simulink model "MCVT_Integration"  (R2020a)
settings: Solver=ode8  StartTime=0.0  StopTime=T_end  FixedStep=dt  RelTol=1e-3  EnableMultiTasking=on
values from neighbouring files (symbols this model uses):
  assumed_power_voltage = 1.25e2    (= 125)    [MCVT_Input_File.m]    (also in MCVT_Run_QuickCheck.m)
  battery_power_toggle = 1    [MCVT_Input_File.m]    (also in MCVT_Run_QuickCheck.m)
  battery_trickle_charge_param = 0.1    [MCVT_Input_File.m]    (also in MCVT_Run_QuickCheck.m)
  battery_trickle_leakage_param = 0.01    [MCVT_Input_File.m]    (also in MCVT_Run_QuickCheck.m)
  damage_case_id = 3    [MCVT_Input_File.m]    (also in MCVT_Run_QuickCheck.m = 1)
  dt = 1/fs    (= 0.001)    [MCVT_Run_QuickCheck.m]    (also in MCVT_Sim_Set.m)
  dt_2 = 1/fs_2    (= 0.001)    [MCVT_Run_QuickCheck.m]    (also in MCVT_Sim_Set.m)
  dt_3 = 1/fs_3    (= 0.001)    [MCVT_Run_QuickCheck.m]    (also in MCVT_Sim_Set.m)
  dt_5 = 1/fs_5    (= 0.005)    [MCVT_Run_QuickCheck.m]    (also in MCVT_Sim_Set.m)
  dt_8 = 1/fs_8    (= 0.05)    [MCVT_Run_QuickCheck.m]    (also in MCVT_Sim_Set.m)
  dt_9 = 1/fs_9    (= 0.001)    [MCVT_Run_QuickCheck.m]    (also in MCVT_Sim_Set.m)
  dust_met_duration = 3    [MCVT_Input_File.m]    (also in MCVT_Run_QuickCheck.m = 2)
  ECLSS_indoor_set_temperature = 298.15    [MCVT_Input_File.m]    (also in MCVT_Run_QuickCheck.m)
  energy_store_max_o = 150    [MCVT_Input_File.m]    (also in MCVT_Run_QuickCheck.m)
  energy_store_o = 120    [MCVT_Input_File.m]    (also in MCVT_Run_QuickCheck.m)
  fs = 1000    [MCVT_Run_QuickCheck.m]    (also in MCVT_Sim_Set.m)
  gain_charge_param = 1    [MCVT_Input_File.m]    (also in MCVT_Run_QuickCheck.m)
  ground_temperature = 373    [MCVT_Habitat_Design_Input_File.m]    (also in MCVT_Run_QuickCheck.m)
  I_max_charge = 800    [MCVT_Input_File.m]    (also in MCVT_Run_QuickCheck.m)
  I_max_discharge = 800    [MCVT_Input_File.m]    (also in MCVT_Run_QuickCheck.m)
  initial_pressure = 1e5    (= 100000)    [MCVT_Input_File.m]    (also in MCVT_Run_QuickCheck.m)
  initial_temperature = 295    [MCVT_Input_File.m]    (also in MCVT_Run_QuickCheck.m)
  interior_leak_rate = 0.00415    [MCVT_Input_File.m]    (also in MCVT_Run_QuickCheck.m)
  launch_dust_accum_start_time = 10    [MCVT_Input_File.m]    (also in MCVT_Run_QuickCheck.m = 2)
  launch_dust_accum_stop_time = 12    [MCVT_Input_File.m]    (also in MCVT_Run_QuickCheck.m = 3)
  meteorite_impact_time = 2    [MCVT_Input_File.m]    (also in MCVT_Run_QuickCheck.m = 1)
  nuclear_power_toggle = 1    [MCVT_Input_File.m]    (also in MCVT_Run_QuickCheck.m)
  print_interval = 30    [MCVT_Input_File.m]
  SOC_lower_thres = 0.5    [MCVT_Input_File.m]    (also in MCVT_Run_QuickCheck.m)
  SOC_upper_thres = 0.9    [MCVT_Input_File.m]    (also in MCVT_Run_QuickCheck.m)
  solar_power_toggle = 1    [MCVT_Input_File.m]    (also in MCVT_Run_QuickCheck.m)
  space_temperature = 4    [MCVT_Habitat_Design_Input_File.m]    (also in MCVT_Run_QuickCheck.m)
  Start_angle = 90    [MCVT_Input_File.m]    (also in MCVT_Run_QuickCheck.m)
undefined symbols (not defined in the file or in its folder): A_flow_restrictor, ACTIVITY_LOOKUP_TABLE, air, Aref, Bmap, Bmap2, Bmap3, comms, DiscIntNeverNeededParam, DmgSPL, dust_parameters, ECLSS_dust_damage_repair_time, ECLSS_heater_capacity, ECLSS_IPS_restrictor, ECLSS_IPS_setpointPressure, ECLSS_paint_damage_repair_time, ECLSS_radiatorLoop_discreteNumber, ECLSS_Vst, emptyActivity, expected_heat_add, FI, habitat, HIEM2SMM, HIEM2STM, HIEMDamageElements, impact_numbering, initial_SPLT_damage_index, input_air, input_Cond, input_CP, input_discretization, input_EV, input_Evap, input_radiatorLoop, K_ECLSS_dust_single, K_ECLSS_paint_single, K_Structure_damage_single, Kref, kW_to_W, meteoroidDustDep, mFRF, ndelays, nominalDustDep, noverlap, nuclear_toggle_gain, output_limits, panel_paint0, Ps0, reference_temperature, RepairTime_ECLSS_factor, Sensor_Location_SMM, SMM_T0, SMM_T1, SMM_T2, SMM_T3, SMM_T4, SMM_T4_0, SMM_T4_1, SMM_T4_2, solar_FDD_K ... (+73)
libraries: neural x350, simulink x39, xpcutilitieslib x7, xpcrtudplib x7, dspstat3 x1
(part 18 of 43)

== system MCVT_Integration/Sys2 - Structural System/Sys2_Structural_System/Fault Detection and Diagnosis (FDD) with Binary Classifer/Pattern Recognition Neural Network/Segment 5/Process Output 1 ==
  a : Inport  PortDimensions=2
      mask Display: e = 0.06;c1 = [120 230 180]/255;c2 = ([120 230 180]+0)/(256+128);c3 = ([120 230 180]+128)/(256+128);patch([0 1 1 0],[0 0 1 1],c1);patch([0 1 1 1-e 1-e 0+e],[0 0 1 1-e 0+e 0+e],c2);patch([0 0 1 1-e 0+e 0+e],[0 1 1 1-e 1-e 0+e],c3);plot([0 1 1 0 0],[0 0 1 1 0]);
  y : Outport  PortDimensions=2
      mask Display: e = 0.06;c1 = [120 230 180]/255;c2 = ([120 230 180]+0)/(256+128);c3 = ([120 230 180]+128)/(256+128);patch([0 1 1 0],[0 0 1 1],c1);patch([0 1 1 1-e 1-e 0+e],[0 0 1 1-e 0+e 0+e],c2);patch([0 0 1 1-e 0+e 0+e],[0 1 1 1-e 1-e 0+e],c3);plot([0 1 1 0 0],[0 0 1 1 0]);
  wires:
  a/1 -> y/1

== system MCVT_Integration/Sys2 - Structural System/Sys2_Structural_System/Fault Detection and Diagnosis (FDD) with Binary Classifer/Subsystem/Enabled Subsystem3/Pattern Recognition Neural Network1 ==
  Input : Inport  PortDimensions=45  SampleTime=0.001
      mask Display: e = 0.06;c1 = [120 230 180]/255;c2 = ([120 230 180]+0)/(256+128);c3 = ([120 230 180]+128)/(256+128);patch([0 1 1 0],[0 0 1 1],c1);patch([0 1 1 1-e 1-e 0+e],[0 0 1 1-e 0+e 0+e],c2);patch([0 0 1 1-e 0+e 0+e],[0 1 1 1-e 1-e 0+e],c3);plot([0 1 1 0 0],[0 0 1 1 0]);
  Layer 1 : Subsystem
      mask Display: e = 0.06;c1 = [120 150 230]/255;c2 = ([120 150 230]+0)/383;c3 = ([120 150 230]+128)/383;patch([0 1 1 0],[0 0 1 1],c1);patch([0 1 1 1-e 1-e 0+e],[0 0 1 1-e 0+e 0+e],c2);patch([0 0 1 1-e 0+e 0+e],[0 1 1 1-e 1-e 0+e],c3);plot([0 1 1 0 0],[0 0 1 1 0]);
  Layer 2 : Subsystem
      mask Display: e = 0.06;c1 = [120 150 230]/255;c2 = ([120 150 230]+0)/383;c3 = ([120 150 230]+128)/383;patch([0 1 1 0],[0 0 1 1],c1);patch([0 1 1 1-e 1-e 0+e],[0 0 1 1-e 0+e 0+e],c2);patch([0 0 1 1-e 0+e 0+e],[0 1 1 1-e 1-e 0+e],c3);plot([0 1 1 0 0],[0 0 1 1 0]);
  Layer 3 : Subsystem
      mask Display: e = 0.06;c1 = [120 150 230]/255;c2 = ([120 150 230]+0)/383;c3 = ([120 150 230]+128)/383;patch([0 1 1 0],[0 0 1 1],c1);patch([0 1 1 1-e 1-e 0+e],[0 0 1 1-e 0+e 0+e],c2);patch([0 0 1 1-e 0+e 0+e],[0 1 1 1-e 1-e 0+e],c3);plot([0 1 1 0 0],[0 0 1 1 0]);
  Layer 4 : Subsystem
      mask Display: e = 0.06;c1 = [120 150 230]/255;c2 = ([120 150 230]+0)/383;c3 = ([120 150 230]+128)/383;patch([0 1 1 0],[0 0 1 1],c1);patch([0 1 1 1-e 1-e 0+e],[0 0 1 1-e 0+e 0+e],c2);patch([0 0 1 1-e 0+e 0+e],[0 1 1 1-e 1-e 0+e],c3);plot([0 1 1 0 0],[0 0 1 1 0]);
  Process Input 1 : Subsystem
      mask Display: e = 0.06;c1 = [120 150 230]/255;c2 = ([120 150 230]+0)/383;c3 = ([120 150 230]+128)/383;patch([0 1 1 0],[0 0 1 1],c1);patch([0 1 1 1-e 1-e 0+e],[0 0 1 1-e 0+e 0+e],c2);patch([0 0 1 1-e 0+e 0+e],[0 1 1 1-e 1-e 0+e],c3);plot([0 1 1 0 0],[0 0 1 1 0]);
  Process Output 1 : Subsystem
      mask Display: e = 0.06;c1 = [120 150 230]/255;c2 = ([120 150 230]+0)/383;c3 = ([120 150 230]+128)/383;patch([0 1 1 0],[0 0 1 1],c1);patch([0 1 1 1-e 1-e 0+e],[0 0 1 1-e 0+e 0+e],c2);patch([0 0 1 1-e 0+e 0+e],[0 1 1 1-e 1-e 0+e],c3);plot([0 1 1 0 0],[0 0 1 1 0]);
  Output : Outport  InitialOutput=[0;0;0;0;0]
      mask Display: e = 0.06;c1 = [120 230 180]/255;c2 = ([120 230 180]+0)/(256+128);c3 = ([120 230 180]+128)/(256+128);patch([0 1 1 0],[0 0 1 1],c1);patch([0 1 1 1-e 1-e 0+e],[0 0 1 1-e 0+e 0+e],c2);patch([0 0 1 1-e 0+e 0+e],[0 1 1 1-e 1-e 0+e],c3);plot([0 1 1 0 0],[0 0 1 1 0]);
  wires:
  Input/1 -> Process Input 1/1(x)
  Process Output 1/1(y) -> Output/1
  Process Input 1/1(p) -> Layer 1/1("p{1}")
  Layer 1/1("a{1}") -> [feedback1]
  [feedback1] -> Layer 2/1("a{1} ")
  Layer 2/1("a{2}") -> [feedback2]
  [feedback2] -> Layer 3/1("a{2} ")
  Layer 3/1("a{3}") -> [feedback3]
  [feedback3] -> Layer 4/1("a{3} ")
  Layer 4/1("a{4}") -> Process Output 1/1(a)

== system MCVT_Integration/Sys2 - Structural System/Sys2_Structural_System/Structural Thermal Model 2.2/STM Temperature Sensors/ Resistance Temperature Detector/RTD with Self-heat Error ==
  Resistance Change with Error : Inport
  " Current in Circuit" : Constant  Value=0.001
  MATLAB Function : MATLAB Function  TreatAsAtomicUnit=on
  Voltage Output with Error : Outport  VectorParamsAs1DForOutWhenUnconnected=off
  wires:
  Resistance Change with Error/1 -> MATLAB Function/2(R)
  MATLAB Function/1(U) -> Voltage Output with Error/1
  " Current in Circuit"/1 -> MATLAB Function/1(I)

== system MCVT_Integration/Sys2 - Structural System/Sys2_Structural_System/Structural Thermal Model 2.2/STM Temperature Sensors/ Resistance Temperature Detector/RTD without Self-heat Error ==
  Resistance Change without Error : Inport
  " Current in Circuit" : Constant  Value=0.001
  MATLAB Function : MATLAB Function  TreatAsAtomicUnit=on
  Current in Circuit : Outport  VectorParamsAs1DForOutWhenUnconnected=off
  Voltage Output without Error : Outport  Port=2  VectorParamsAs1DForOutWhenUnconnected=off
  wires:
  Resistance Change without Error/1 -> MATLAB Function/2(R)
  MATLAB Function/2(U) -> Voltage Output without Error/1
  MATLAB Function/1(I) -> Current in Circuit/1
  " Current in Circuit"/1 -> MATLAB Function/1("I ")

== system MCVT_Integration/Sys2 - Structural System/Sys2_Structural_System/Structural Thermal Model 2.2/STM Temperature Sensors/ Resistance Temperature Detector/Self-heat Calculation ==
  note: Power = I 2 *R
  Real Temperature : Inport
  Resistance Change without Error : Inport  Port=2
  Current in Circuit : Inport  Port=3
  "  T vs R " : MATLAB Function  TreatAsAtomicUnit=on
  Add1 : Sum
  Divide1 : Product  Inputs=*/
  Gain : Gain  Gain=1000
  Product : Product
  Self Heating Constant (mW/℃) : Constant  Value=50
  Square : Math  Operator=square  SignedPower=on  SaturateOnIntegerOverflow=on
  Resistance Change with Error : Outport  VectorParamsAs1DForOutWhenUnconnected=off
  Self-heat Error : Outport  Port=2  VectorParamsAs1DForOutWhenUnconnected=off
  wires:
  Self Heating Constant (mW/℃)/1 -> Divide1/2
  Product/1 -> Divide1/1
  Add1/1 -> "  T vs R "/1(T)
  Divide1/1 -> Self-heat Error/1, Add1/2
  Resistance Change without Error/1 -> Product/1
  "  T vs R "/1(R) -> Resistance Change with Error/1
  Real Temperature/1 -> Add1/1
  Gain/1 -> Square/1
  Square/1 -> Product/2
  Current in Circuit/1 -> Gain/1

== system MCVT_Integration/Sys2 - Structural System/Sys2_Structural_System/Structural Thermal Model 2.2/STM Temperature Sensors/ Resistance Temperature Detector/Switch ON//OFF Control Block ==
  In1 : Inport
  In2 : Inport  Port=2
  Multiport Switch1 : MultiPortSwitch  Inputs=2
  Switch OFF : Subsystem
  Switch ON : Subsystem
  Out1 : Outport  VectorParamsAs1DForOutWhenUnconnected=off
  wires:
  Multiport Switch1/1 -> Out1/1
  In2/1 -> Switch ON/1(In1), Switch OFF/1(In1)
  In1/1 -> Multiport Switch1/1
  Switch ON/1(ON) -> Multiport Switch1/2
  Switch OFF/1(OFF) -> Multiport Switch1/3

== system MCVT_Integration/Sys2 - Structural System/Sys2_Structural_System/Structural Thermal Model 2.2/STM Temperature Sensors/ Resistance Temperature Detector1/RTD with Self-heat Error ==
  Resistance Change with Error : Inport
  " Current in Circuit" : Constant  Value=0.001
  MATLAB Function : MATLAB Function  TreatAsAtomicUnit=on
  Voltage Output with Error : Outport  VectorParamsAs1DForOutWhenUnconnected=off
  wires:
  " Current in Circuit"/1 -> MATLAB Function/1(I)
  MATLAB Function/1(U) -> Voltage Output with Error/1
  Resistance Change with Error/1 -> MATLAB Function/2(R)

== system MCVT_Integration/Sys2 - Structural System/Sys2_Structural_System/Structural Thermal Model 2.2/STM Temperature Sensors/ Resistance Temperature Detector1/RTD without Self-heat Error ==
  Resistance Change without Error : Inport
  " Current in Circuit" : Constant  Value=0.001
  MATLAB Function : MATLAB Function  TreatAsAtomicUnit=on
  Current in Circuit : Outport  VectorParamsAs1DForOutWhenUnconnected=off
  Voltage Output without Error : Outport  Port=2  VectorParamsAs1DForOutWhenUnconnected=off
  wires:
  " Current in Circuit"/1 -> MATLAB Function/1("I ")
  MATLAB Function/1(I) -> Current in Circuit/1
  MATLAB Function/2(U) -> Voltage Output without Error/1
  Resistance Change without Error/1 -> MATLAB Function/2(R)

== system MCVT_Integration/Sys2 - Structural System/Sys2_Structural_System/Structural Thermal Model 2.2/STM Temperature Sensors/ Resistance Temperature Detector1/Self-heat Calculation ==
  note: Power = I 2 *R
  Real Temperature : Inport
  Resistance Change without Error : Inport  Port=2
  Current in Circuit : Inport  Port=3
  "  T vs R " : MATLAB Function  TreatAsAtomicUnit=on
  Add1 : Sum
  Divide1 : Product  Inputs=*/
  Gain : Gain  Gain=1000
  Product : Product
  Self Heating Constant (mW/℃) : Constant  Value=50
  Square : Math  Operator=square  SignedPower=on  SaturateOnIntegerOverflow=on
  Resistance Change with Error : Outport  VectorParamsAs1DForOutWhenUnconnected=off
  Self-heat Error : Outport  Port=2  VectorParamsAs1DForOutWhenUnconnected=off
  wires:
  Current in Circuit/1 -> Gain/1
  Square/1 -> Product/2
  Gain/1 -> Square/1
  Real Temperature/1 -> Add1/1
  "  T vs R "/1(R) -> Resistance Change with Error/1
  Resistance Change without Error/1 -> Product/1
  Divide1/1 -> Add1/2, Self-heat Error/1
  Add1/1 -> "  T vs R "/1(T)
  Product/1 -> Divide1/1
  Self Heating Constant (mW/℃)/1 -> Divide1/2

== system MCVT_Integration/Sys2 - Structural System/Sys2_Structural_System/Structural Thermal Model 2.2/STM Temperature Sensors/ Resistance Temperature Detector1/Switch ON//OFF Control Block ==
  In1 : Inport
  In2 : Inport  Port=2
  Multiport Switch1 : MultiPortSwitch  Inputs=2
  Switch OFF : Subsystem
  Switch ON : Subsystem
  Out1 : Outport  VectorParamsAs1DForOutWhenUnconnected=off
  wires:
  Switch OFF/1(OFF) -> Multiport Switch1/3
  Switch ON/1(ON) -> Multiport Switch1/2
  In1/1 -> Multiport Switch1/1
  In2/1 -> Switch OFF/1(In1), Switch ON/1(In1)
  Multiport Switch1/1 -> Out1/1

== system MCVT_Integration/Sys2 - Structural System/Sys2_Structural_System/Structural Thermal Model 2.2/STM Temperature Sensors/ Resistance Temperature Detector2/RTD with Self-heat Error ==
  Resistance Change with Error : Inport
  " Current in Circuit" : Constant  Value=0.001
  MATLAB Function : MATLAB Function  TreatAsAtomicUnit=on
  Voltage Output with Error : Outport  VectorParamsAs1DForOutWhenUnconnected=off
  wires:
  Resistance Change with Error/1 -> MATLAB Function/2(R)
  MATLAB Function/1(U) -> Voltage Output with Error/1
  " Current in Circuit"/1 -> MATLAB Function/1(I)

== system MCVT_Integration/Sys2 - Structural System/Sys2_Structural_System/Structural Thermal Model 2.2/STM Temperature Sensors/ Resistance Temperature Detector2/RTD without Self-heat Error ==
  Resistance Change without Error : Inport
  " Current in Circuit" : Constant  Value=0.001
  MATLAB Function : MATLAB Function  TreatAsAtomicUnit=on
  Current in Circuit : Outport  VectorParamsAs1DForOutWhenUnconnected=off
  Voltage Output without Error : Outport  Port=2  VectorParamsAs1DForOutWhenUnconnected=off
  wires:
  Resistance Change without Error/1 -> MATLAB Function/2(R)
  MATLAB Function/2(U) -> Voltage Output without Error/1
  MATLAB Function/1(I) -> Current in Circuit/1
  " Current in Circuit"/1 -> MATLAB Function/1("I ")

== system MCVT_Integration/Sys2 - Structural System/Sys2_Structural_System/Structural Thermal Model 2.2/STM Temperature Sensors/ Resistance Temperature Detector2/Self-heat Calculation ==
  note: Power = I 2 *R
  Real Temperature : Inport
  Resistance Change without Error : Inport  Port=2
  Current in Circuit : Inport  Port=3
  "  T vs R " : MATLAB Function  TreatAsAtomicUnit=on
  Add1 : Sum
  Divide1 : Product  Inputs=*/
  Gain : Gain  Gain=1000
  Product : Product
  Self Heating Constant (mW/℃) : Constant  Value=50
  Square : Math  Operator=square  SignedPower=on  SaturateOnIntegerOverflow=on
  Resistance Change with Error : Outport  VectorParamsAs1DForOutWhenUnconnected=off
  Self-heat Error : Outport  Port=2  VectorParamsAs1DForOutWhenUnconnected=off
  wires:
  Self Heating Constant (mW/℃)/1 -> Divide1/2
  Product/1 -> Divide1/1
  Add1/1 -> "  T vs R "/1(T)
  Divide1/1 -> Self-heat Error/1, Add1/2
  Resistance Change without Error/1 -> Product/1
  "  T vs R "/1(R) -> Resistance Change with Error/1
  Real Temperature/1 -> Add1/1
  Gain/1 -> Square/1
  Square/1 -> Product/2
  Current in Circuit/1 -> Gain/1

== system MCVT_Integration/Sys2 - Structural System/Sys2_Structural_System/Structural Thermal Model 2.2/STM Temperature Sensors/ Resistance Temperature Detector2/Switch ON//OFF Control Block ==
  In1 : Inport
  In2 : Inport  Port=2
  Multiport Switch1 : MultiPortSwitch  Inputs=2
  Switch OFF : Subsystem
  Switch ON : Subsystem
  Out1 : Outport  VectorParamsAs1DForOutWhenUnconnected=off
  wires:
  Multiport Switch1/1 -> Out1/1
  In2/1 -> Switch ON/1(In1), Switch OFF/1(In1)
  In1/1 -> Multiport Switch1/1
  Switch ON/1(ON) -> Multiport Switch1/2
  Switch OFF/1(OFF) -> Multiport Switch1/3

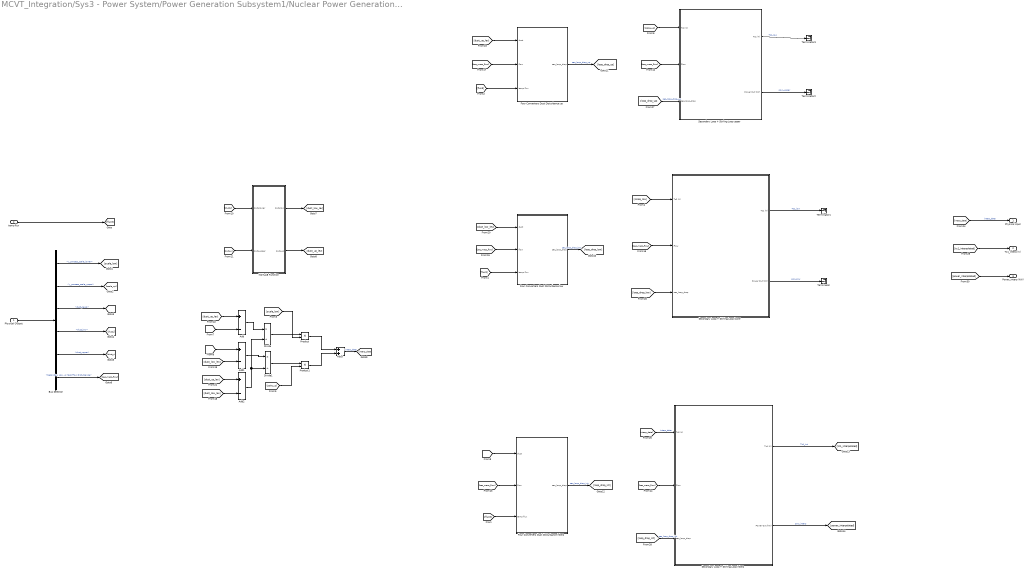
== system MCVT_Integration/Sys3 - Power System/Power Generation Subsystem1/Nuclear Power Generation/SP-100 Power Generation/	Power Generation/Interpolation ==
  Physical Output : Inport
  temp flux : Inport  Port=2
  Add : Sum  Inputs=+-
  Add1 : Sum  Inputs=+-
  Add2 : Sum  Inputs=+-
  Add3 : Sum
  Bus Selector : BusSelector  OutputSignals=y_process_scale_lower,y_process_scale_upper,dust_level,dust_low,dust_upper,Secondary Loop Mass Flow Disturbance
  Divide : Product  Inputs=*/
  Divide1 : Product  Inputs=*/
  Four Converters Dust Disturbance interp : Subsystem
  Four Converters Dust Disturbance low : Subsystem
  Four Converters Dust Disturbance up : Subsystem
  MATLAB Function : MATLAB Function  TreatAsAtomicUnit=on
  Product : Product
  Product1 : Product
  Secondary Loop + Stirling Loop interp : Subsystem
  Secondary Loop + Stirling Loop lower : Subsystem
  Secondary Loop + Stirling Loop upper : Subsystem
  Terminator{,1..3} (4 alike) : Terminator
  Physical Input : Outport
  Tc1_interp (K) : Outport  Port=2
  Power_interp (kW) : Outport  Port=3
  wires:
  Product/1 -> Add3/1
  [dust_l] -> MATLAB Function/1(dust_lower)
  Add1/1 -> Divide1/1
  [dust_u] -> MATLAB Function/2(dust_upper)
  Product1/1 -> Add3/2
  [interp_data] -> Physical Input/1  "interp_data"
  Divide1/1 -> Product1/1
  Divide/1 -> Product/1
  Add/1 -> Divide/1
  [dust_low_lev] -> Add2/2
  [scale_up] -> Product1/2
  [scale_low] -> Product/2
  Physical Output/1 -> Bus Selector/1
  Add2/1 -> Divide/2, Divide1/2
  [dust_up_lev] -> Add2/1
  [d_level] -> Add/2
  [sec_mass_flow] -> Secondary Loop + Stirling Loop upper/2(Flow)
  Secondary Loop + Stirling Loop interp/1(Tc1 (K)) -> [tc1_interpolated]  "Tc1_int"
  Secondary Loop + Stirling Loop interp/2(Power Out (kW)) -> [power_interpolated]  "pow_interp"
  Secondary Loop + Stirling Loop lower/2(Power Out (kW)) -> Terminator/1  "pow_low"
  Secondary Loop + Stirling Loop lower/1(Tc1 (K)) -> Terminator1/1  "Tc1_low"
  Secondary Loop + Stirling Loop upper/2(Power Out (kW)) -> Terminator2/1  "pow_upper"
  Secondary Loop + Stirling Loop upper/1(Tc1 (K)) -> Terminator3/1  "Tc1_low"
  [flux3] -> Four Converters Dust Disturbance interp/3(temp flux)
  Four Converters Dust Disturbance low/1(sec_loop_drop) -> [loop_drop_low]  "sec_loop_drop_low"
  [loop_drop_up] -> Secondary Loop + Stirling Loop upper/3(sec_loop_drop)  "sec_loop_drop_up"
  [loop_drop_int] -> Secondary Loop + Stirling Loop interp/3(sec_loop_drop)  "sec_loop_drop_int"
  temp flux/1 -> [flux3]
  [flux3] -> Four Converters Dust Disturbance low/3(temp flux)
  [flux3] -> Four Converters Dust Disturbance up/3(temp flux)
  Bus Selector/1 -> [scale_low]  "<y_process_scale_lower>"
  [scale_low] -> Secondary Loop + Stirling Loop lower/1(Tc2 (K))
  Bus Selector/2 -> [scale_up]  "<y_process_scale_upper>"
  [scale_up] -> Secondary Loop + Stirling Loop upper/1(Tc2 (K))
  Bus Selector/3 -> [d_level]  "<dust_level>"
  [d_level] -> Four Converters Dust Disturbance interp/1(dust)
  [d_level] -> Add1/1
  Bus Selector/4 -> [dust_l]  "<dust_low>"
  Bus Selector/5 -> [dust_u]  "<dust_upper>"
  Bus Selector/6 -> [sec_mass_flow]  "<Secondary Loop Mass Flow Disturbance>"
  [sec_mass_flow] -> Four Converters Dust Disturbance interp/2(flow)
  [sec_mass_flow] -> Secondary Loop + Stirling Loop interp/2(Flow)
  [sec_mass_flow] -> Four Converters Dust Disturbance low/2(flow)
  [sec_mass_flow] -> Secondary Loop + Stirling Loop lower/2(Flow)
  [sec_mass_flow] -> Four Converters Dust Disturbance up/2(flow)
  MATLAB Function/1(dust_low) -> [dust_low_lev]
  [dust_low_lev] -> Add1/2
  [dust_low_lev] -> Four Converters Dust Disturbance low/1(dust)
  MATLAB Function/2(dust_up) -> [dust_up_lev]
  [dust_up_lev] -> Add/1
  [dust_up_lev] -> Four Converters Dust Disturbance up/1(dust)
  Add3/1 -> [interp_data]  "interp_data"
  [interp_data] -> Secondary Loop + Stirling Loop interp/1(Tc2 (K))  "interp_data"
  [loop_drop_low] -> Secondary Loop + Stirling Loop lower/3(sec_loop_drop)
  Four Converters Dust Disturbance up/1(sec_loop_drop) -> [loop_drop_up]  "sec_loop_drop_up"
  Four Converters Dust Disturbance interp/1(sec_loop_drop) -> [loop_drop_int]  "sec_loop_drop_int"
  [tc1_interpolated] -> Tc1_interp (K)/1
  [power_interpolated] -> Power_interp (kW)/1

== system MCVT_Integration/Sys3 - Power System/Power Generation Subsystem1/Nuclear Power Generation/SP-100 Power Generation/	Power Generation/Nominal Power ==
  nominal values : Inport
  temp flux : Inport  Port=2
  flow : Inport  Port=3
  Constant19 : Constant  Value=[1 2 3 5]
  Constant2 : Constant  Value=output_limits
  Four Converters Dust Disturbance : Subsystem
  Secondary Loop + Stirling Loop : Subsystem
  Sensor Models : Subsystem
  nominal dust : Constant
  scale [-1,1] to process value5 : MATLAB Function  TreatAsAtomicUnit=on
  Measured Temp Values Nominal (K) : Outport
  power nominal (kW) : Outport  Port=2
  wires:
  Constant19/1 -> scale [-1,1] to process value5/3(inds)
  Constant2/1 -> scale [-1,1] to process value5/2(output_limits)
  nominal values/1 -> [nominal_values]
  [y_scale_nom] -> Secondary Loop + Stirling Loop/1(y_process_scale_lower)  "y_process_scale_nom"
  temp flux/1 -> [flux_var]
  [flow2] -> Secondary Loop + Stirling Loop/2(Flow)
  Four Converters Dust Disturbance/1(sec_loop_drop) -> [sec_drop]  "sec_loop_drop_nom"
  Secondary Loop + Stirling Loop/1(Tc1_lower) -> [tc1_nominal]  "Tc1_nom"
  Secondary Loop + Stirling Loop/2(Power Out) -> [power_out_nom]  "power_nom"
  Sensor Models/1(Cyber Output Nom) -> Measured Temp Values Nominal (K)/1
  nominal dust/1 -> Four Converters Dust Disturbance/1(dust)
  Sensor Models/2(Physical Output SP100 Nom) -> power nominal (kW)/1
  [nominal_values] -> scale [-1,1] to process value5/1(y_unitscale)
  [flux_var] -> Four Converters Dust Disturbance/3(temp flux)
  flow/1 -> [flow2]
  [flow2] -> Four Converters Dust Disturbance/2(flow)
  [sec_drop] -> Secondary Loop + Stirling Loop/3(sec_loop_drop)
  scale [-1,1] to process value5/1(y_process_scale_lower) -> [y_scale_nom]  "y_process_scale_nom"
  [y_scale_nom] -> Sensor Models/1(Physical Input)  "y_process_scale_nom"
  [tc1_nominal] -> Sensor Models/3(tc1)
  [power_out_nom] -> Sensor Models/2(power)

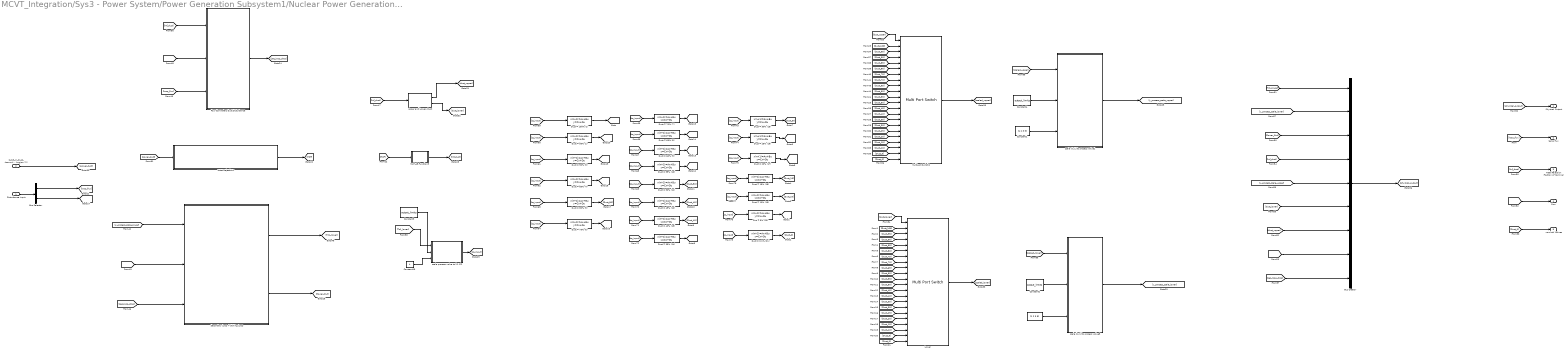
== system MCVT_Integration/Sys3 - Power System/Power Generation Subsystem1/Nuclear Power Generation/SP-100 Power Generation/	Power Generation/Power Generation ==
  Disturbance Input : Inport
  Sub3_In_Dust_Accumulation (mg/cm^2) : Inport  Port=2
  " " : Constant  Value=[1 2 3 5]
  Bus Creator : BusCreator  Inputs=9
  Bus Selector : BusSelector  OutputSignals=secondary loop fluctuation,Secondary Loop Mass Flow Disturbance
  Constant1 : Constant  Value=[1 2 3 5]
  Constant15 : Constant  Value=4
  Constant2 : Constant  Value=output_limits
  Constant3 : Constant  Value=output_limits
  Constant4 : Constant  Value=output_limits
  Dus = 5% (20) : DiscreteStateSpace  A=sys_n4sid_20.A  B=sys_n4sid_20.B  C=sys_n4sid_20.C  D=sys_n4sid_20.D  InitialCondition=X0_20
  Dust = 0.1% (21) : DiscreteStateSpace  A=sys_n4sid_21.A  B=sys_n4sid_21.B  C=sys_n4sid_21.C  D=sys_n4sid_21.D  InitialCondition=X0_21
  Dust = 10% (19) : DiscreteStateSpace  A=sys_n4sid_19.A  B=sys_n4sid_19.B  C=sys_n4sid_19.C  D=sys_n4sid_19.D  InitialCondition=X0_19
  Dust = 100% (1) : DiscreteStateSpace  A=sys_n4sid_1.A  B=sys_n4sid_1.B  C=sys_n4sid_1.C  D=sys_n4sid_1.D  InitialCondition=X0_1
  Dust = 15% (18) : DiscreteStateSpace  A=sys_n4sid_18.A  B=sys_n4sid_18.B  C=sys_n4sid_18.C  D=sys_n4sid_18.D  InitialCondition=X0_18
  Dust = 20% (17) : DiscreteStateSpace  A=sys_n4sid_17.A  B=sys_n4sid_17.B  C=sys_n4sid_17.C  D=sys_n4sid_17.D  InitialCondition=X0_17
  Dust = 25% (16) : DiscreteStateSpace  A=sys_n4sid_16.A  B=sys_n4sid_16.B  C=sys_n4sid_16.C  D=sys_n4sid_16.D  InitialCondition=X0_16
  Dust = 30% (15) : DiscreteStateSpace  A=sys_n4sid_15.A  B=sys_n4sid_15.B  C=sys_n4sid_15.C  D=sys_n4sid_15.D  InitialCondition=X0_15
  Dust = 35% (14) : DiscreteStateSpace  A=sys_n4sid_14.A  B=sys_n4sid_14.B  C=sys_n4sid_14.C  D=sys_n4sid_14.D  InitialCondition=X0_14
  Dust = 40% (13) : DiscreteStateSpace  A=sys_n4sid_13.A  B=sys_n4sid_13.B  C=sys_n4sid_13.C  D=sys_n4sid_13.D  InitialCondition=X0_13
  Dust = 45% (12) : DiscreteStateSpace  A=sys_n4sid_12.A  B=sys_n4sid_12.B  C=sys_n4sid_12.C  D=sys_n4sid_12.D  InitialCondition=X0_12
  Dust = 50% (11) : DiscreteStateSpace  A=sys_n4sid_11.A  B=sys_n4sid_11.B  C=sys_n4sid_11.C  D=sys_n4sid_11.D  InitialCondition=X0_11
  Dust = 55% (10) : DiscreteStateSpace  A=sys_n4sid_10.A  B=sys_n4sid_10.B  C=sys_n4sid_10.C  D=sys_n4sid_10.D  InitialCondition=X0_10
  Dust = 60% (9) : DiscreteStateSpace  A=sys_n4sid_9.A  B=sys_n4sid_9.B  C=sys_n4sid_9.C  D=sys_n4sid_9.D  InitialCondition=X0_9
  Dust = 65% (8) : DiscreteStateSpace  A=sys_n4sid_8.A  B=sys_n4sid_8.B  C=sys_n4sid_8.C  D=sys_n4sid_8.D  InitialCondition=X0_8
  Dust = 70% (7) : DiscreteStateSpace  A=sys_n4sid_7.A  B=sys_n4sid_7.B  C=sys_n4sid_7.C  D=sys_n4sid_7.D  InitialCondition=X0_7
  Dust = 75% (6) : DiscreteStateSpace  A=sys_n4sid_6.A  B=sys_n4sid_6.B  C=sys_n4sid_6.C  D=sys_n4sid_6.D  InitialCondition=X0_6
  Dust = 80% (5) : DiscreteStateSpace  A=sys_n4sid_5.A  B=sys_n4sid_5.B  C=sys_n4sid_5.C  D=sys_n4sid_5.D  InitialCondition=X0_5
  Dust = 85% (4) : DiscreteStateSpace  A=sys_n4sid_4.A  B=sys_n4sid_4.B  C=sys_n4sid_4.C  D=sys_n4sid_4.D  InitialCondition=X0_4
  Dust = 90% (3) : DiscreteStateSpace  A=sys_n4sid_3.A  B=sys_n4sid_3.B  C=sys_n4sid_3.C  D=sys_n4sid_3.D  InitialCondition=X0_3
  Dust = 95% (2) : DiscreteStateSpace  A=sys_n4sid_2.A  B=sys_n4sid_2.B  C=sys_n4sid_2.C  D=sys_n4sid_2.D  InitialCondition=X0_2
  Four Converters Dust Disturbance : Subsystem
  Heat Rejection1 : Subsystem
  Lower : MultiPortSwitch  Inputs=21
  MATLAB Function3 : MATLAB Function  TreatAsAtomicUnit=on
  Multiport Switch2 : MultiPortSwitch  Inputs=21
  Secondary Loop + Stirling Loop : Subsystem
  Upoer and Lowew Dust : MATLAB Function  TreatAsAtomicUnit=on
  scale [-1,1] to process value1 : MATLAB Function  TreatAsAtomicUnit=on
  scale [-1,1] to process value5 : MATLAB Function  TreatAsAtomicUnit=on
  scale process value to [-1,1]2 : MATLAB Function  TreatAsAtomicUnit=on
  Physical Output : Outport
  temp flux : Outport  Port=2
  Heat Rejection Fraction of Nominal : Outport  Port=3
  flow : Outport  Port=4
  nominal values : Outport  Port=5
  wires:
  Disturbance Input/1 -> Bus Selector/1
  [loop_flux] -> temp flux/1
  [sec_loop_drop] -> Secondary Loop + Stirling Loop/3(sec_loop_drop)  "sec_loop_drop"
  Secondary Loop + Stirling Loop/1(Tc1_lower) -> [Tc1_lower]  "Tc1_low"
  Constant15/1 -> scale process value to [-1,1]2/3(ind)
  Dust = 100% (1)/1 -> [Dust_100]  "dust100"
  Dust = 95% (2)/1 -> [Dust_95]  "dust95"
  Dust = 90% (3)/1 -> [Dust_90]
  Dust = 85% (4)/1 -> [Dust_85]
  Dust = 80% (5)/1 -> [Dust_80]
  Dust = 75% (6)/1 -> [Dust_75]
  Dust = 70% (7)/1 -> [Dust_70]
  Dust = 65% (8)/1 -> [Dust_65]
  Dust = 60% (9)/1 -> [Dust_60]
  Dust = 50% (11)/1 -> [Dust_50]
  Dust = 20% (17)/1 -> [Dust_20]
  Dust = 30% (15)/1 -> [Dust_30]
  Dust = 15% (18)/1 -> [Dust_15]
  Dust = 10% (19)/1 -> [Dust_10]
  Dus = 5% (20)/1 -> [Dust_5]
  Dust = 0.1% (21)/1 -> [Dust_0]
  Dust = 35% (14)/1 -> [Dust_35]
  Dust = 40% (13)/1 -> [Dust_40]
  Dust = 45% (12)/1 -> [Dust_45]
  Dust = 55% (10)/1 -> [Dust_55]
  [Dust_70] -> Lower/8
  [Dust_5] -> Lower/21
  [Dust_10] -> Lower/20
  [Dust_45] -> Lower/13
  [Dust_80] -> Lower/6
  [Dust_75] -> Lower/7
  [Dust_55] -> Lower/11
  [Dust_15] -> Lower/19
  [Dust_85] -> Lower/5
  [Dust_35] -> Lower/15
  [Dust_95] -> Lower/3
  [Dust_60] -> Lower/10
  [Dust_25] -> Lower/17
  [Dust_90] -> Lower/4
  [Dust_20] -> Lower/18
  [Dust_100] -> Lower/2
  [Dust_30] -> Lower/16
  [Dust_40] -> Lower/14
  [Dust_0] -> Lower/22
  [Dust_50] -> Lower/12
  [Dust_65] -> Lower/9
  " "/1 -> scale [-1,1] to process value5/3(inds)
  Lower/1 -> [scaled_lower]  "scaled_lower"
  Constant2/1 -> scale [-1,1] to process value5/2(output_limits)
  scale [-1,1] to process value5/1(y_process_scale_lower) -> [y_process_scale_lower]  "y_process_scale_lower"
  [hrf_dust] -> Bus Creator/4  "dust_level"
  Secondary Loop + Stirling Loop/2(Power Out) -> [Power_Out]
  [Power_Out] -> Bus Creator/3  "power_out"
  [Tc1_lower] -> Bus Creator/1  "tc1_lower"
  Dust = 25% (16)/1 -> [Dust_25]
  Constant3/1 -> scale process value to [-1,1]2/2(output_limits)
  [y_process_scale_lower] -> Secondary Loop + Stirling Loop/1(y_process_scale_lower)
  Bus Creator/1 -> [physical_output]
  [hrf_dust] -> Upoer and Lowew Dust/1(hrf)  "dust_intervention"
  Heat Rejection1/1(Heat Rejection Fraction) -> [HRF]  "heat_rejection_fraction_nominal"
  Sub3_In_Dust_Accumulation (mg/cm^2)/1 -> [power_dust]
  [Dust_20] -> Multiport Switch2/18
  scale [-1,1] to process value1/1(y_process_scale_upper) -> [y_process_scale_upper]  "y_process_scale_upper"
  Constant4/1 -> scale [-1,1] to process value1/2(output_limits)
  [Dust_85] -> Multiport Switch2/5
  [Dust_60] -> Multiport Switch2/10
  [Dust_100] -> Multiport Switch2/2
  Multiport Switch2/1 -> [scaled_upper]  "scaled_lower"
  [Dust_30] -> Multiport Switch2/16
  [Dust_90] -> Multiport Switch2/4
  [Dust_35] -> Multiport Switch2/15
  [Dust_55] -> Multiport Switch2/11
  [dust_upper] -> Bus Creator/7  "dust_upper"
  [Dust_70] -> Multiport Switch2/8
  [Dust_80] -> Multiport Switch2/6
  [Dust_95] -> Multiport Switch2/3
  [Dust_65] -> Multiport Switch2/9
  [Dust_40] -> Multiport Switch2/14
  [Dust_75] -> Multiport Switch2/7
  [Dust_5] -> Multiport Switch2/21
  [Dust_50] -> Multiport Switch2/12
  [Dust_0] -> Multiport Switch2/22
  [Dust_15] -> Multiport Switch2/19
  [Dust_45] -> Multiport Switch2/13
  [Dust_25] -> Multiport Switch2/17
  [Dust_10] -> Multiport Switch2/20
  Constant1/1 -> scale [-1,1] to process value1/3(inds)
  [dust_lower] -> Lower/1  "dust_low"
  [HRF] -> MATLAB Function3/1(hrf_in)  "dust_intervention"
  Bus Selector/1 -> [loop_flux]  "<secondary loop fluctuation>"
  [loop_flux] -> Four Converters Dust Disturbance/3(temp flux)
  Bus Selector/2 -> [mass_flow]  "<Secondary Loop Mass Flow Disturbance>"
  [mass_flow] -> Bus Creator/8
  [mass_flow] -> flow/1
  [mass_flow] -> Secondary Loop + Stirling Loop/2(Flow)
  [mass_flow] -> Four Converters Dust Disturbance/2(flow)
  MATLAB Function3/1(hrf_out) -> [hrf_dust]  "dust_intervention"
  [hrf_dust] -> Four Converters Dust Disturbance/1(dust)  "dust_intervention"
  [hrf_dust] -> Heat Rejection Fraction of Nominal/1  "dust_intervention"
  Four Converters Dust Disturbance/1(sec_loop_drop) -> [sec_loop_drop]  "sec_loop_drop"
  [sec_loop_drop] -> Bus Creator/9  "sec_loop_drop"
  [Tc1_lower] -> scale process value to [-1,1]2/1(u)
  scale process value to [-1,1]2/1(y) -> [ss_input]
  [ss_input] -> Dust = 100% (1)/1
  [ss_input] -> Dust = 95% (2)/1
  [ss_input] -> Dust = 90% (3)/1
  [ss_input] -> Dust = 85% (4)/1
  [ss_input] -> Dust = 80% (5)/1
  [ss_input] -> Dust = 75% (6)/1
  [ss_input] -> Dust = 70% (7)/1
  [ss_input] -> Dust = 65% (8)/1
  [ss_input] -> Dust = 60% (9)/1
  [ss_input] -> Dust = 55% (10)/1
  [ss_input] -> Dust = 50% (11)/1
  [ss_input] -> Dust = 45% (12)/1
  [ss_input] -> Dust = 40% (13)/1
  [ss_input] -> Dust = 35% (14)/1
  [ss_input] -> Dust = 30% (15)/1
  [ss_input] -> Dust = 25% (16)/1
  [ss_input] -> Dust = 20% (17)/1
  [ss_input] -> Dust = 15% (18)/1
  [ss_input] -> Dust = 10% (19)/1
  [ss_input] -> Dus = 5% (20)/1
  [ss_input] -> Dust = 0.1% (21)/1
  [Dust_0] -> nominal values/1
  [power_dust] -> Heat Rejection1/1(dust_accumulation_in mg/cm^2)
  Upoer and Lowew Dust/1(dust_up) -> [dust_upper]  "dust_upper"
  [dust_upper] -> Multiport Switch2/1  "dust_upper"
  Upoer and Lowew Dust/2(dust_low) -> [dust_lower]  "dust_low"
  [dust_lower] -> Bus Creator/6  "dust_low"
  [scaled_lower] -> scale [-1,1] to process value5/1(y_unitscale)
  [y_process_scale_lower] -> Bus Creator/2
  [scaled_upper] -> scale [-1,1] to process value1/1(y_unitscale)
  [y_process_scale_upper] -> Bus Creator/5
  [physical_output] -> Physical Output/1

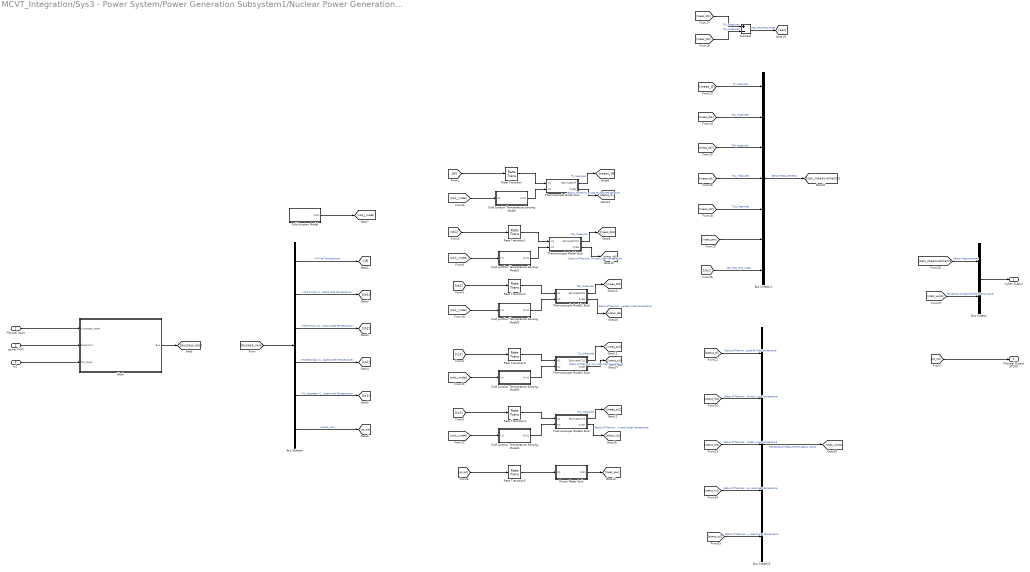
== system MCVT_Integration/Sys3 - Power System/Power Generation Subsystem1/Nuclear Power Generation/SP-100 Power Generation/	Power Generation/Sensor Models ==
  Physical Input : Inport
  power (kW) : Inport  Port=2
  tc1 : Inport  Port=3
  Bus Creator : BusCreator  Inputs=2
  Bus Creator1 : BusCreator  Inputs=7
  Bus Creator3 : BusCreator  Inputs=5
  Bus Selector : BusSelector  OutputSignals=TF Fuel Temperature,Tb1 Primary Coolant Inlet Temperature,Tb2 Primary Coolant Outlet Temperature,Tc2 Secondary Coolant Outlet Temperature,Tc1 Secondary Coolant Inlet Temperature,power_out
  Cold Junction Model : Subsystem
  Cold Junction Temperature Sensing Model : Subsystem
  Cold Junction Temperature Sensing Model1 : Subsystem
  Cold Junction Temperature Sensing Model2 : Subsystem
  Cold Junction Temperature Sensing Model3 : Subsystem
  Cold Junction Temperature Sensing Model4 : Subsystem
  Power Meter Stub : Subsystem
  Rate Transition{,1..5} (6 alike) : RateTransition  OutPortSampleTime=dt_3
  Subtract : Sum  Inputs=+-
  Thermocouple Mode Stub : Subsystem
  Thermocouple Model Stub : Subsystem
  Thermocouple Model2 Stub : Subsystem
  Thermocouple Model3 Stub : Subsystem
  Thermocouple Model4 Stub : Subsystem
  block : Subsystem
  Cyber Output : Outport
  Physical Output SP100 : Outport  Port=2
  wires:
  [tf] -> Rate Transition/1
  [meas_tf] -> Bus Creator1/1  "Tf_measured"
  Cold Junction Temperature Sensing Model/1(Out1) -> Thermocouple Mode Stub/2(In2)
  [meas_tb1] -> Bus Creator1/2  "Tb1_measured"
  [tb1] -> Rate Transition1/1
  [meas_tb2] -> Bus Creator1/3  "Tb2_measured"
  [tb2] -> Rate Transition2/1
  [cold_j_model] -> Cold Junction Temperature Sensing Model/1(In1)
  Cold Junction Temperature Sensing Model2/1(Out1) -> Thermocouple Model2 Stub/2(In2)
  Cold Junction Temperature Sensing Model1/1(Out1) -> Thermocouple Model Stub/2(In2)
  Cold Junction Temperature Sensing Model4/1(Out1) -> Thermocouple Model4 Stub/2(In2)
  Cold Junction Temperature Sensing Model3/1(Out1) -> Thermocouple Model3 Stub/2(In2)
  [sen_measurements] -> Bus Creator/1  "Sensor Measurements"
  [meas_tc1] -> Bus Creator1/4  "Tc1_measured"
  [meas_tc2] -> Bus Creator1/5  "Tc2_measured"
  Bus Creator/1 -> Cyber Output/1
  block/1(Bus) -> [bussed_vals]
  [tc1] -> Rate Transition4/1
  [status_tf] -> Bus Creator3/1  "Status of Thermocouple for Fuel Temperature"
  [status_tb1] -> Bus Creator3/2  "Status of Thermocouple for Primary Loop Temperature"
  [status_tb2] -> Bus Creator3/3  "Status of Thermocouple for Avg. Secondary Loop Temperature"
  [status_tc1] -> Bus Creator3/4  "Status of Thermocouple for Secondary Loop Inlet Temperature"
  [status_tc2] -> Bus Creator3/5  "Status of Thermocouple for Secondary Loop Outlet Temperature"
  [stats_words] -> Bus Creator/2  "Temperature Measurement Status Words"
  [sec] -> Bus Creator1/7  "sec_loop_drop_meas"
  [meas_pow] -> Bus Creator1/6
  Physical Input/1 -> block/1(y_process_scale)
  [tc2] -> Rate Transition3/1
  Rate Transition/1 -> Thermocouple Mode Stub/1(In1)
  Rate Transition1/1 -> Thermocouple Model Stub/1(In1)
  Rate Transition2/1 -> Thermocouple Model2 Stub/1(In1)
  Rate Transition3/1 -> Thermocouple Model3 Stub/1(In1)
  Rate Transition4/1 -> Thermocouple Model4 Stub/1(In1)
  Rate Transition5/1 -> Power Meter Stub/1(In1)
  [po_out] -> Rate Transition5/1
  power (kW)/1 -> block/2(Power Out)
  tc1/1 -> block/3(Tc1_lower)
  [bussed_vals] -> Bus Selector/1
  Bus Selector/1 -> [tf]  "<TF Fuel Temperature>"
  Bus Selector/2 -> [tb1]  "<Tb1 Primary Coolant Inlet Temperature>"
  Bus Selector/3 -> [tb2]  "<Tb2 Primary Coolant Outlet Temperature>"
  Bus Selector/4 -> [tc2]  "<Tc2 Secondary Coolant Outlet Temperature>"
  Bus Selector/5 -> [tc1]  "<Tc1 Secondary Coolant Inlet Temperature>"
  Bus Selector/6 -> [po_out]  "<power_out>"
  [po_out] -> Physical Output SP100/1
  Cold Junction Model/1(Out1) -> [cold_j_model]
  [cold_j_model] -> Cold Junction Temperature Sensing Model1/1(In1)
  [cold_j_model] -> Cold Junction Temperature Sensing Model2/1(In1)
  [cold_j_model] -> Cold Junction Temperature Sensing Model3/1(In1)
  [cold_j_model] -> Cold Junction Temperature Sensing Model4/1(In1)
  Thermocouple Mode Stub/1(Estimated Tf) -> [meas_tf]  "Tf_measured"
  Thermocouple Model Stub/1(Estimated Tb1) -> [meas_tb1]  "Tb1_measured"
  Thermocouple Model2 Stub/1(Estimated Tb2) -> [meas_tb2]  "Tb2_measured"
  Thermocouple Model3 Stub/1(Estimated Tc1) -> [meas_tc1]  "Tc1_measured"
  [meas_tc1] -> Subtract/1  "Tc1_measured"
  Thermocouple Model4 Stub/1(Estimated Tc2) -> [meas_tc2]  "Tc2_measured"
  [meas_tc2] -> Subtract/2  "Tc2_measured"
  Power Meter Stub/1(Out1) -> [meas_pow]
  Thermocouple Mode Stub/2(Cyber) -> [status_tf]  "Status of Thermocouple for Fuel Temperature"
  Thermocouple Model Stub/2(Cyber) -> [status_tb1]  "Status of Thermocouple for Primary Loop Temperature"
  Thermocouple Model2 Stub/2(Cyber) -> [status_tb2]  "Status of Thermocouple for Avg. Secondary Loop Temperature"
  Thermocouple Model3 Stub/2(Cyber) -> [status_tc1]  "Status of Thermocouple for Secondary Loop Inlet Temperature"
  Thermocouple Model4 Stub/2(Cyber) -> [status_tc2]  "Status of Thermocouple for Secondary Loop Outlet Temperature"
  Subtract/1 -> [sec]  "sec_loop_drop_meas"
  Bus Creator3/1 -> [stats_words]  "Temperature Measurement Status Words"
  Bus Creator1/1 -> [sen_measurements]  "Sensor Measurements"

== system MCVT_Integration/Sys3 - Power System/Power Generation Subsystem1/Nuclear Power Generation/SP-100 Power Generation/FDD Iterative Online Residual Analysis1/ Digital Twin of SP100 ==
  Physical Output SP100 : Inport
  power nominal (kW) : Inport  Port=2
  Sub3_In_Intervention : Inport  Port=3
  Residual Generation : Subsystem
  Cyber Output FDD : Outport
  wires:
  Residual Generation/1(Cyber Output) -> Cyber Output FDD/1
  power nominal (kW)/1 -> Residual Generation/2(power nominal (kW))
  Physical Output SP100/1 -> Residual Generation/1(Physical Output SP100)
  Sub3_In_Intervention/1 -> Residual Generation/3(Sub3_In_Intervention)

== system MCVT_Integration/Sys8 - Interior Environment/Habitat Interior Environment Model/Temperature Sensors/ Resistance Temperature Detector/Switch ON//OFF Control Block/Switch OFF ==
  In1 : Inport
  Clock1 : Clock
  MATLAB Function1 : MATLAB Function  TreatAsAtomicUnit=on  SystemSampleTime=dt_8
  No Signal : Scope
  OFF : Outport  VectorParamsAs1DForOutWhenUnconnected=off
  wires:
  In1/1 -> MATLAB Function1/1(u)
  Clock1/1 -> MATLAB Function1/2(Time)
  MATLAB Function1/1(y) -> OFF/1, No Signal/1

== system MCVT_Integration/Sys8 - Interior Environment/Habitat Interior Environment Model/Temperature Sensors/ Resistance Temperature Detector/Switch ON//OFF Control Block/Switch ON ==
  In1 : Inport
  Gain2 : Gain
  ON : Outport  VectorParamsAs1DForOutWhenUnconnected=off
  wires:
  Gain2/1 -> ON/1
  In1/1 -> Gain2/1

== system MCVT_Integration/Sys8 - Interior Environment/Habitat Interior Environment Model/Temperature Sensors/ Resistance Temperature Detector1/Switch ON//OFF Control Block/Switch OFF ==
  In1 : Inport
  Clock1 : Clock
  MATLAB Function1 : MATLAB Function  TreatAsAtomicUnit=on  SystemSampleTime=dt_8
  No Signal : Scope
  OFF : Outport  VectorParamsAs1DForOutWhenUnconnected=off
  wires:
  In1/1 -> MATLAB Function1/1(u)
  Clock1/1 -> MATLAB Function1/2(Time)
  MATLAB Function1/1(y) -> OFF/1, No Signal/1

== system MCVT_Integration/Sys8 - Interior Environment/Habitat Interior Environment Model/Temperature Sensors/ Resistance Temperature Detector1/Switch ON//OFF Control Block/Switch ON ==
  In1 : Inport
  Gain2 : Gain
  Signal Received : Scope
  ON : Outport  VectorParamsAs1DForOutWhenUnconnected=off
  wires:
  Gain2/1 -> ON/1, Signal Received/1
  In1/1 -> Gain2/1

== system MCVT_Integration/Sys8 - Interior Environment/Habitat Interior Environment Model/Temperature Sensors/ Resistance Temperature Detector2/Switch ON//OFF Control Block/Switch OFF ==
  In1 : Inport
  Clock1 : Clock
  MATLAB Function1 : MATLAB Function  TreatAsAtomicUnit=on  SystemSampleTime=dt_8
  No Signal : Scope
  OFF : Outport  VectorParamsAs1DForOutWhenUnconnected=off
  wires:
  In1/1 -> MATLAB Function1/1(u)
  Clock1/1 -> MATLAB Function1/2(Time)
  MATLAB Function1/1(y) -> OFF/1, No Signal/1

== system MCVT_Integration/Sys8 - Interior Environment/Habitat Interior Environment Model/Temperature Sensors/ Resistance Temperature Detector2/Switch ON//OFF Control Block/Switch ON ==
  In1 : Inport
  Gain2 : Gain
  Signal Received : Scope
  ON : Outport  VectorParamsAs1DForOutWhenUnconnected=off
  wires:
  Gain2/1 -> ON/1, Signal Received/1
  In1/1 -> Gain2/1

== system MCVT_Integration/Sys2 - Structural System/Sys2_Structural_System/Fault Detection and Diagnosis (FDD) 2.3/Accelerometer/Accelerometer/Switch ON//OFF Control Block/Switch OFF ==
  Sensor : Inport
  All Acc : Inport  Port=2
  Clock1 : Clock
  MATLAB Function1 : MATLAB Function  TreatAsAtomicUnit=on
  Selected Acc : Outport  VectorParamsAs1DForOutWhenUnconnected=off
  wires:
  Sensor/1 -> MATLAB Function1/1(sensor)
  MATLAB Function1/1(y) -> Selected Acc/1
  Clock1/1 -> MATLAB Function1/3(Time)
  All Acc/1 -> MATLAB Function1/2(u)

== system MCVT_Integration/Sys2 - Structural System/Sys2_Structural_System/Fault Detection and Diagnosis (FDD) 2.3/Accelerometer/Accelerometer/Switch ON//OFF Control Block/Switch ON ==
  In1 : Inport
  Gain2 : Gain
  Signal Received : Scope
  ON : Outport  VectorParamsAs1DForOutWhenUnconnected=off
  wires:
  In1/1 -> Gain2/1
  Gain2/1 -> Signal Received/1, ON/1

== system MCVT_Integration/Sys2 - Structural System/Sys2_Structural_System/Fault Detection and Diagnosis (FDD) 2.3/Pattern Recognition Neural Network/Pattern Recognition Neural Network with 10% Noise/Layer 1/Delays 1 ==
  "p{1}" : Inport  PortDimensions=2304
      mask Display: e = 0.06;c1 = [120 230 180]/255;c2 = ([120 230 180]+0)/(256+128);c3 = ([120 230 180]+128)/(256+128);patch([0 1 1 0],[0 0 1 1],c1);patch([0 1 1 1-e 1-e 0+e],[0 0 1 1-e 0+e 0+e],c2);patch([0 0 1 1-e 0+e 0+e],[0 1 1 1-e 1-e 0+e],c3);plot([0 1 1 0 0],[0 0 1 1 0]);
  mux : Mux  Inputs=1
      mask Display: e = 0.06;c1 = [120 150 230]/255;c2 = ([120 150 230]+0)/383;c3 = ([120 150 230]+128)/383;patch([0 1 1 0],[0 0 1 1],c1);patch([0 1 1 1-e 1-e 0+e],[0 0 1 1-e 0+e 0+e],c2);patch([0 0 1 1-e 0+e 0+e],[0 1 1 1-e 1-e 0+e],c3);plot([0 1 1 0 0],[0 0 1 1 0]);disp('Mux')
  "pd{1,1}" : Outport
      mask Display: e = 0.06;c1 = [120 230 180]/255;c2 = ([120 230 180]+0)/(256+128);c3 = ([120 230 180]+128)/(256+128);patch([0 1 1 0],[0 0 1 1],c1);patch([0 1 1 1-e 1-e 0+e],[0 0 1 1-e 0+e 0+e],c2);patch([0 0 1 1-e 0+e 0+e],[0 1 1 1-e 1-e 0+e],c3);plot([0 1 1 0 0],[0 0 1 1 0]);
  wires:
  "p{1}"/1 -> mux/1
  mux/1 -> "pd{1,1}"/1
